simulink model "SerialPractice"  (R2017a)
settings: Solver=FixedStepAuto  StartTime=0.0  StopTime=10  FixedStep=0.01  MinStep=0.001  RelTol=1e-3  EnableMultiTasking=on
model workspace (MAT data in the model file):
  A = -0.291988550171
  B = -3.35930459163
  BladeGeometry = [0.0525 0.08 0.488692190558 0.0575 0.0765 0.471238898038 0.0625 0.073 0.453785605519 0.0675 0.0695 0.436332312999 ... (102 elements, 3x34)]
  MCOS : class17 (value not decoded)
  C = 1
  CMCoeff = [0.0123861263102 0.0100869558121 0.00395957088672 0.0120046459416 0.000145393148265 0.000524554028707 -0.00381348366885 -0.000510658981973 -0.000304808013498 2.16037945221e-05 0.000267649913084 4.61550112894e-05 ... (15 elements, 1x15)]
  Cd = 0
  CpCoeff = [0.0934076671766 -0.182695655179 -0.0130608968061 0.168650159118 0.0246505329395 0.00117759523912 -0.0369025055261 -0.00816552386632 -0.000573147306806 5.84997495063e-05 0.00238658647448 0.000659038696901 ... (15 elements, 1x15)]
  CpMat = [0.00867983704985 0.0104119952175 0.0123710198164 0.0145730804094 0.0170242990451 0.0197435241026 0.0227463845937 0.0260322039195 0.0296166959289 0.0335240991161 0.0377450541312 0.0423117873146 ... (4998 elements, 98x51)]
  CpMat_Iter4 = [-0.0174922752096 -0.0192000701213 -0.0208598028222 -0.0224761244863 -0.0240370794106 -0.0255486645229 -0.0270020954202 -0.0283906118498 -0.0297102509597 -0.0309617439636 -0.0321423489123 -0.0332305675829 ... (1116 elements, 31x36)]
  CqMat = [0.00950017223156 0.0100359591617 0.0106270141471 0.0112699479611 0.0119664102911 0.012716418653 0.0135224725245 0.0143788698852 0.0152889983241 0.0162542310373 0.017269427342 0.0183409321549 ... (4998 elements, 98x51)]
  CqMat_Iter4 = [-0.00651761334335 -0.00659827180546 -0.00666156814481 -0.00671038770655 -0.00674205252964 -0.00676213602267 -0.00676868409106 -0.00675993762446 -0.00673707609481 -0.00670413785211 -0.00666096044769 -0.00660422169135 ... (1116 elements, 31x36)]
  CtMat = [0.288467055178 0.29651503174 0.305320968805 0.314902207207 0.325269269239 0.336435131701 0.348427518089 0.36126603808 0.374993913486 0.389643061676 0.405260174906 0.421894254361 ... (4998 elements, 98x51)]
  CtMat_Iter4 = [0.270159937237 0.280676295654 0.292081972796 0.304364398908 0.317529220148 0.331577864133 0.346520948014 0.362369170866 0.379136481365 0.396833726898 0.415486832803 0.435128972133 ... (1116 elements, 31x36)]
  D = 0
  F = 8.99888284546
  G1 = 3.42440748358
  G2 = 0.707106781187
  GenPowerMat = [2.1012 9.4178 21.4169168091 35.1462940199 48.8871910299 67.2083870432 1.98778255814 9.28771731844 21.0754826816 34.5144 47.9983864865 65.9770351351 ... (48 elements, 6x8)]
  GenTorqMat = [0.0501624549637 0.119911153844 0.185924082325 0.239730275341 0.274610756692 0.305615629004 0.047454812988 0.118254889702 0.182960031645 0.235420172908 0.269618134227 0.300016322066 ... (48 elements, 6x8)]
  MCOS : class17 (value not decoded)
  K = -0.496937064767
  MCOS : class17 (value not decoded)
  MCOS : class17 (value not decoded)
  MCOS : class17 (value not decoded)
  MCOS : class17 (value not decoded)
  MCOS : class17 (value not decoded)
  MCOS : class17 (value not decoded)
  MCOS : class17 (value not decoded)
  MCOS : class17 (value not decoded)
  MCOS : class17 (value not decoded)
  MCOS : class17 (value not decoded)
  MCOS : class17 (value not decoded)
  PowerMat = [0 0.000462072097155 0.000925921899779 0.00139155621296 0.00185898187069 0.00232820573596 0.00279923470096 0.00327207568717 0.00374673564551 0.00422322155651 0.00470154043042 0.00518169930735 ... (3512982 elements, 6666x17x31)]
  MCOS : class17 (value not decoded)
  MCOS : class17 (value not decoded)
  MCOS : class17 (value not decoded)
  MCOS : class17 (value not decoded)
  MCOS : class17 (value not decoded)
  MCOS : class17 (value not decoded)
  MCOS : class17 (value not decoded)
  MCOS : class17 (value not decoded)
  MCOS : class17 (value not decoded)
  MCOS : class17 (value not decoded)
  MCOS : class17 (value not decoded)
  MCOS : class17 (value not decoded)
  RecEtaMat = [0.532050517794 0.640021818962 0.683186617187 0.705089035203 0.72694096499 0.756076871372 0.556339856293 0.649839345247 0.690435804048 0.714712461207 0.738846952049 0.771026273171 ... (48 elements, 6x8)]
  MCOS : class17 (value not decoded)
  TorqueMat = [0.0188980666332 0.0189200731218 0.0189421220361 0.0189642134844 0.0189863475752 0.0190085244173 0.01903074412 0.0190530067929 0.0190753125458 0.0190976614891 0.0191200537334 0.0191424893896 ... (3512982 elements, 6666x17x31)]
  V_r = 11
  MCOS : class17 (value not decoded)
  MCOS : class17 (value not decoded)
  MCOS : class17 (value not decoded)
  bladeRpmVec = [309.48670493 412.998876697 516.511048465 620.023220232 723.535392 827.047563768 930.559735535 1034.0719073 1137.58407907 1241.09625084 1344.60842261 1448.12059437 ... (41 elements, 1x41)]
  bladeTorqueMat = [0.00679712928319 0.00852288742527 0.0105717008499 0.012902132331 0.0154507893622 0.0182235621523 0.021227269718 0.0244699016413 0.0279609406934 0.0317118022158 0.0409095986767 0.0494145988338 ... (656 elements, 16x41)]
  break1_Cp = [1 2 3 4 5 6 7 8 9 10 11 12 ... (98 elements, 1x98)]
  break1_Cp_Iter4 = [1 1.1 1.2 1.3 1.4 1.5 1.6 1.7 1.8 1.9 2 2.1 ... (31 elements, 1x31)]
  break1_Cq = [1 2 3 4 5 6 7 8 9 10 11 12 ... (98 elements, 1x98)]
  break1_Cq_Iter4 = [1 1.1 1.2 1.3 1.4 1.5 1.6 1.7 1.8 1.9 2 2.1 ... (31 elements, 1x31)]
  break1_Ct = [1 2 3 4 5 6 7 8 9 10 11 12 ... (98 elements, 1x98)]
  break1_Ct_Iter4 = [1 1.1 1.2 1.3 1.4 1.5 1.6 1.7 1.8 1.9 2 2.1 ... (31 elements, 1x31)]
  break2_Cp = [1 2 3 4 5 6 7 8 9 10 11 12 ... (51 elements, 1x51)]
  break2_Cp_Iter4 = [-15 -14 -13 -12 -11 -10 -9 -8 -7 -6 -5 -4 ... (36 elements, 1x36)]
  break2_Cq = [1 2 3 4 5 6 7 8 9 10 11 12 ... (51 elements, 1x51)]
  break2_Cq_Iter4 = [-15 -14 -13 -12 -11 -10 -9 -8 -7 -6 -5 -4 ... (36 elements, 1x36)]
  break2_Ct = [1 2 3 4 5 6 7 8 9 10 11 12 ... (51 elements, 1x51)]
  break2_Ct_Iter4 = [-15 -14 -13 -12 -11 -10 -9 -8 -7 -6 -5 -4 ... (36 elements, 1x36)]
  dpitch = 1
  dpitch_Iter4 = 1
  dtsr = 0.1
  dtsr_Iter4 = 0.1
  generatorRpmVec = [341.915107735 356.773957938 376.905565127 404.234314097 443.280769879 505.264428386 745.052922197 1327.1653456 1680.18103026 1897.48535507 2373.16400484 2811.26212639 ... (16 elements, 16x1)]
  generatorTorqueVec = [0.0238551497253 0.030635265767 0.0389685483873 0.0489528429291 0.0610820639797 0.076486398825 0.111942081576 0.216661798023 0.273917248647 0.300910880899 0.342762941842 0.368779609659 ... (16 elements, 16x1)]
  loadBreak = [0.5 0.6 0.8 1 1.3 1.5 2 3]
  MCOS : class17 (value not decoded)
  oBreak = [0 0.103201266712 0.206402533425 0.309603800137 0.412805066849 0.516006333561 0.619207600274 0.722408866986 0.825610133698 0.928811400411 1.03201266712 1.13521393384 ... (6666 elements, 1x6666)]
  MCOS : class17 (value not decoded)
  p = -2.67879336333
  pBreak = [0 1 2 3 4 5 6 7 8 9 10 11 ... (31 elements, 1x31)]
  pitchIn = [-10 -9 -8 -7 -6 -5 -4 -3 -2 -1 0 1 ... (41 elements, 41x1)]
  pitchMat = [-10 -10 -10 -10 -10 -10 -10 -10 -10 -10 -10 -10 ... (656 elements, 16x41)]
  pitchMat_Cp = [1 1 1 1 1 1 1 1 1 1 1 1 ... (4998 elements, 98x51)]
  pitchMat_Cp_Iter4 = [-15 -14 -13 -12 -11 -10 -9 -8 -7 -6 -5 -4 ... (36 elements, 1x36)]
  pitchMat_Cq = [1 1 1 1 1 1 1 1 1 1 1 1 ... (4998 elements, 98x51)]
  pitchMat_Cq_Iter4 = [-15 -14 -13 -12 -11 -10 -9 -8 -7 -6 -5 -4 ... (1116 elements, 36x31)]
  pitchMat_Ct = [1 1 1 1 1 1 1 1 1 1 1 1 ... (4998 elements, 98x51)]
  pitchMat_Ct_Iter4 = [-15 -14 -13 -12 -11 -10 -9 -8 -7 -6 -5 -4 ... (1116 elements, 36x31)]
  pitchOpt = [13 14 13 9 7 4 3 10 15 18 21 23 ... (16 elements, 16x1)]
  pitchVec = [-10 -9 -8 -7 -6 -5 -4 -3 -2 -1 0 1 ... (41 elements, 41x1)]
  pitch_Start = -10
  pitch_Start_Iter4 = -15
  pitch_r = 11
  MCOS : class17 (value not decoded)
  rpmBreak = [400 750 1100 1400 1700 2100]
  rpmMat = [309.48670493 312.485093221 316.121075916 320.361079054 325.130275341 330.482781466 336.483551241 343.211141152 350.761447592 359.252856498 381.925342476 405.594424779 ... (656 elements, 16x41)]
  tsrMat_Cp = [1 1.1 1.2 1.3 1.4 1.5 1.6 1.7 1.8 1.9 2 2.1 ... (4998 elements, 98x51)]
  tsrMat_Cp_Iter4 = [1 1.1 1.2 1.3 1.4 1.5 1.6 1.7 1.8 1.9 2 2.1 ... (31 elements, 1x31)]
  tsrMat_Cq = [1 1.1 1.2 1.3 1.4 1.5 1.6 1.7 1.8 1.9 2 2.1 ... (4998 elements, 98x51)]
  tsrMat_Cq_Iter4 = [1 1 1 1 1 1 1 1 1 1 1 1 ... (1116 elements, 36x31)]
  tsrMat_Ct = [1 1.1 1.2 1.3 1.4 1.5 1.6 1.7 1.8 1.9 2 2.1 ... (4998 elements, 98x51)]
  tsrMat_Ct_Iter4 = [1 1 1 1 1 1 1 1 1 1 1 1 ... (1116 elements, 36x31)]
  MCOS : class17 (value not decoded)
  tsr_Start = 1
  tsr_Start_Iter4 = 1
  vBreak = [5 6 7 8 9 10 11 12 13 14 15 16 ... (17 elements, 1x17)]
  windIn = [5 6 7 8 9 10 11 12 13 14 15 16 ... (16 elements, 16x1)]
  windMat = [5 6 7 8 9 10 11 12 13 14 15 16 ... (656 elements, 16x41)]
  windVec = [5 6 7 8 9 10 11 12 13 14 15 16 ... (16 elements, 16x1)]
undefined symbols (not defined in the file or in its folder): LF, serial
libraries: instrumentlib x3
(part 2 of 2)

== matlab function SerialPractice/To Double ==
  data: ascii input; double output
function double = fcn(ascii)
% Input
%       ascii - number represented in ascii
% Output
%       double - number to be converted
% Assumes each output is length 5:
%       ex: 32.2   = {'3', '2', '.', '3', '0'}
%           0.5    = {'0', '.', '5', '0', '0'}
%           323.7  = {'3', '2', '3' '.', '7'}

if max(ascii) > 0
    n = 6; % Expected number of inputs
    k = 1;
    i = 0;
    
    % Find where data starts
    while k < 2 && i <= n
        i = i + 1;
        if ascii(i) ~= 32 % Find where nulls stop (null = 32)
            k = k+1;
        end
    end
    
    % Get new ascii and new length
    asciiOld = ascii; %#ok
    ascii    = ascii(i:n,1);
    n        = length(ascii);
    
    % Determine if negative in front
    if ascii(1) == 45
        sgn = -1;
        ascii = ascii(2:n,1); % Ignore negative
    else
        sgn = 1;
    end
    
    % Find decimal
    n = length(ascii);
    locDec = find(ascii == 46);
    
    ascii = ascii - 48; % convert to numbers 1-9
    
    % Convert logic
    % locDec = 2 (1.2)   = [(digit 1)*10 + (digit 2)]/10
    % locDec = 3 (11.2)  = [(digit 1)*100 + (digit 2)*10 + (digit 3)]/10
    % locDec = 4 (111.2) = [(digit 1)*1000 + (digit 2)*100 + (digit 3)*10 + (digit 4)]/10
    
    % Convert to double
    doubleVec = zeros(n-1,1);
    for m = 1:n
        if m == locDec % Skip decimal in ascii vector
            m = m + 1; %#ok
        elseif m > locDec % assign decimal to last digit
            doubleVec(m-1) = ascii(m);
        else % Follow convert logic for digits before the decimal
            expn = (locDec-m);
            doubleVec(m) = real(ascii(m)*10^expn);
        end
    end
    
    % Convert logic
    double = sgn*sum(doubleVec)/10;
    
else
    double = 0;
end

== matlab function SerialPractice/To Ascii ==
  data: double input; ascii output
function ascii = fcn(double)
% Input
%       double - number to be converted
% Output
%       ascii - number represented in ascii
% Assumes each output is length 5:
%       ex: 32.2   = {'3', '2', '.', '3', '0'}
%           0.5    = {'0', '.', '5', '0', '0'}
%           323.7  = {'3', '2', '3' '.', '7'}
% Only keep first decimal place
double = double*10;
double = round(double)/10;

% Determine decimal part of input
decPart = (double-floor(double))*10;

% Get sign of double
sgn    = sign(double);
double = abs(double);
k = 1; % order of number (ex: k=2 is a number 10-99)

% Get order of number
doubleCheck = double;
while doubleCheck >= 10 && k < 5
    doubleCheck = doubleCheck/10;
    k = k + 1;
end

% Get individual parts of double
indvNum = zeros(k+1, 1); % Last part is always decimal
doubleNew = floor(double);
for j = 1:k
    % Make left most digit in the tenths decimal place
    doubleNew = doubleNew/10;
    % Get decimal and multiply by to to get digit, store in correct
    % loaction
    indvNum(k-j+1) = (doubleNew - floor(doubleNew))*10;
    % Only keep integer of what's left
    doubleNew = floor(doubleNew);
end

% Last number is the tenths decimal place
indvNum(k+1) = decPart;

n         = 6; % Length of output
ascii     = zeros(n,1); % Initialize ascii output
doub      = [0, 1, 2, 3, 4, 5, 6, 7, 8, 9]; % number equivalent
asc       = [48, 49, 50, 51, 52, 53, 54, 55, 56, 57]; % ascii equivalent

locDec    = k + 1; % Location of decimal
% k = 1 -> decimal place is position 2
% k = 2 -> decimal place is position 3
% k = 3 -> decimal place is position 3

for i = 1:n
    
    if i == locDec % Put a decimal in the appropriate place
        ascii(locDec) = 46; % ascii for decimal
        i = i + 1; %#ok
    elseif i == locDec + 1 % place decimal appropriately
        curr = indvNum(i-1); % Get decimal digit to convert to ascii
        idx = doub == round(curr); % Find row of desired ascii
        ascii(i) = asc(idx); % Store desired ascii
    elseif i > locDec + 1 % Everything after tenths decimal place is zero
        ascii(i) = 0;
    else % convert digit to ascii equivalent
        curr = indvNum(i); % Get current part to convert to ascii
        idx = doub == round(curr); % Find row of desired ascii
        ascii(i) = asc(idx); % Store desired ascii
    end   
end

% add negative sign to beginning of ascii if applicable
if sgn < 0
    ascii(2:n) = ascii(1:n-1);
    ascii(1)   = 45;
end
